simulink model "PrkgClimaEveMgr"  (R2022b) (continued)
== system PrkgClimaEveMgr/PrkgClimaEveMgr_Runnable_sys == (continued)
      settings: EnsureOutportIsVirtual=on
  RtMaxForPrePrkgClima_RtMaxForPrePrkgClima_write : Outport  Port=79
      settings: EnsureOutportIsVirtual=on
  SeatHeatForPrkgClimaEnad_SeatHeatForPrkgClimaEnad_write : Outport  Port=80
      settings: EnsureOutportIsVirtual=on
  SteerWhlHeatgForPrkgClimaEnad_SteerWhlHeatgForPrkgClimaEnad_write : Outport  Port=81
      settings: EnsureOutportIsVirtual=on
  StrtRqrdForDirForPrePrkgClima_StrtRqrdForDirForPrePrkgClima_write : Outport  Port=82
      settings: EnsureOutportIsVirtual=on
  StrtRqrdForPreClng_StrtRqrdForPreClng_write : Outport  Port=83
      settings: EnsureOutportIsVirtual=on
  StrtRqrdForTmrForPrePrkgClima_StrtRqrdForTmrForPrePrkgClima_write : Outport  Port=84
      settings: EnsureOutportIsVirtual=on
  SuspStrtAllwdForPrkgClima_SuspStrtAllwdForPrkgClima_write : Outport  Port=85
      settings: EnsureOutportIsVirtual=on
  TelmClimaExtVentnLvlStrtReq_TelmClimaExtVentnLvlStrtReq_write : Outport  Port=86
      settings: EnsureOutportIsVirtual=on
  TelmClimaExtVentnReqStrtReq_TelmClimaExtVentnReqStrtReq_write : Outport  Port=87
      settings: EnsureOutportIsVirtual=on
  TelmClimaExtVentnTempRangeStrtReq_TelmClimaExtVentnTempRangeStrtReq_write : Outport  Port=88
      settings: EnsureOutportIsVirtual=on
  TelmClimaExtVentnTSpSpclStrtReq_TelmClimaExtVentnTSpSpclStrtReq_write : Outport  Port=89
      settings: EnsureOutportIsVirtual=on
  UpdRtTotForTmrForPrePrkgClima_UpdRtTotForTmrForPrePrkgClima_write : Outport  Port=90
      settings: EnsureOutportIsVirtual=on
  WarnForHybWoU_WarnForHybWoU_write : Outport  Port=91
      settings: EnsureOutportIsVirtual=on
  WarnForHybWoUForTmr_WarnForHybWoUForTmr_write : Outport  Port=92
      settings: EnsureOutportIsVirtual=on
  WarnForPreCdngFctNotAvl_WarnForPreCdngFctNotAvl_write : Outport  Port=93
      settings: EnsureOutportIsVirtual=on
  WarnForTmrPreCdngFctNotAvl_WarnForTmrPreCdngFctNotAvl_write : Outport  Port=94
      settings: EnsureOutportIsVirtual=on
  IntCo2SetStartTime_IntCo2SetStartTime_write : Outport  Port=95
      settings: EnsureOutportIsVirtual=on
  IntCo2SetStopTime_IntCo2SetStopTime_write : Outport  Port=96
      settings: EnsureOutportIsVirtual=on
  RemClimaTempStrtReq_RemClimaTempStrtReq_write : Outport  Port=97
      settings: EnsureOutportIsVirtual=on
  wires:
  AmbTFildForClimaNgcc_AmbTFildForClima_read/1 -> Terminator_1/1
  BookTrvlClimaActvReqfromExsrc_BookTrvlClimaActvReqfromExsrc_read/1 -> Terminator_2/1
  CarTiIntForClima_CarTiIntForClima_read/1 -> Terminator_3/1
  ClimaCfgForCds1_ClimaCfgForCds1_read/1 -> Terminator_4/1
  ClimaCfgForClimaCtrlMan1_ClimaCfgForClimaCtrlMan1_read/1 -> Terminator_5/1
  ClimaCfgForCoolgPreCdn1_ClimaCfgForCoolgPreCdn1_read/1 -> Terminator_6/1
  ClimaCfgForCooltCircForClima1_ClimaCfgForCooltCircForClima1_read/1 -> Terminator_7/1
  ClimaCfgForEcuCooltVlvOutp1_ClimaCfgForEcuCooltVlvOutp1_read/1 -> Terminator_8/1
  ClimaCfgForExtVentnClimaCtrl_ClimaCfgForExtVentnClimaCtrl_read/1 -> Terminator_9/1
  ClimaCfgForFoldCSD_ClimaCfgForFoldCSD_read/1 -> Terminator_10/1
  ClimaCfgForForRemEngStrtPreCdn_ClimaCfgForForRemEngStrtPreCdn_read/1 -> Terminator_11/1
  ClimaCfgForHeatgPreCdnAvl1_ClimaCfgForHeatgPreCdnAvl1_read/1 -> Terminator_12/1
  ClimaCfgForHeatrTyp1_ClimaCfgForHeatrTyp1_read/1 -> Terminator_13/1
  ClimaCfgForHvacHeatrElecFrntPrsnt1_ClimaCfgForHvacHeatrElecFrntPrsnt1_read/1 -> Terminator_14/1
  ClimaCfgForPostCdnAvl1_ClimaCfgForPostCdnAvl1_read/1 -> Terminator_15/1
  ClimaCfgForPreClng1_ClimaCfgForPreClng1_read/1 -> Terminator_16/1
  ClimaCfgForVehElec1_ClimaCfgForVehElec1_read/1 -> Terminator_17/1
  ClimaCfgForVehHyb1_ClimaCfgForVehHyb1_read/1 -> Terminator_18/1
  ClimaCfgForVrntOfVehSysElec1_ClimaCfgForVrntOfVehSysElec1_read/1 -> Terminator_19/1
  ClimaOvrHeatProWarn_ClimaOvrHeatProWarn_read/1 -> Terminator_20/1
  ClimaOvrHeatReqFromExrsc_ClimaOvrHeatReqFromExrsc_read/1 -> Terminator_21/1
  ClimaStrtTiDly_ClimaStrtTiDly_read/1 -> Terminator_22/1
  ClimaTmrSts_ClimaTmrSts_read/1 -> Terminator_23/1
  CmptmtClsd_CmptmtClsd_read/1 -> Terminator_24/1
  COHSts_COHSts_read/1 -> Terminator_25/1
  CooltTForPrkgClima_CooltTForPrkgClima_read/1 -> Terminator_26/1
  DCChrgnHndlSts_DCChrgnHndlSts_read/1 -> Terminator_27/1
  DlyReqForTmrForPrkgClima_DlyReqForTmrForPrkgClima_read/1 -> Terminator_28/1
  DshbTForPrkgClima_DshbTForPrkgClima_read/1 -> Terminator_29/1
  EngT_Ngcc_EngT_read/1 -> Terminator_30/1
  ErsCmdByKeyFromExsrc_ErsCmdByKeyFromExsrc_read/1 -> Terminator_31/1
  ErsRunTimeFromExtSrc_ErsRunTimeFromExtSrc_read/1 -> Terminator_32/1
  HeatrElecNotAvlForTmr_HeatrElecNotAvlForTmr_read/1 -> Terminator_33/1
  HeatrTypSetgForPrkgClima_HeatrTypSetgForPrkgClima_read/1 -> Terminator_34/1
  HeatUpRqrdForPrkgClima_HeatUpRqrdForPrkgClima_read/1 -> Terminator_35/1
  HMIExtVentFanVolReqFromExtSrc_HMIExtVentFanVolReqFromExtSrc_read/1 -> Terminator_36/1
  HMIExtVentonoffReqFromExtSrc_HMIExtVentonoffReqFromExtSrc_read/1 -> Terminator_37/1
  HMIExtVentTSpReqFromExtSrc_HMIExtVentTSpReqFromExtSrc_read/1 -> Terminator_38/1
  HMIExtVentTStsReqFromExtSrc_HMIExtVentTStsReqFromExtSrc_read/1 -> Terminator_39/1
  HvBattSoc_HvBattSoc_read/1 -> Terminator_40/1
  HvSysCnctWoU_HvSysCnctWoU_read/1 -> Terminator_41/1
  HvSysWoU_HvSysWoU_read/1 -> Terminator_42/1
  IntCo2ActvSts_IntCo2ActvSts_read/1 -> Terminator_43/1
  IntCo2fromExsrc_IntCo2fromExsrc_read/1 -> Terminator_44/1
  KeyClimaActv_KeyClimaActv_read/1 -> Terminator_45/1
  LockgCenSts_Ngcc_LockgCenSts_read/1 -> Terminator_46/1
  OnBdChrgrHndlSts_OnBdChrgrHndlSts_read/1 -> Terminator_47/1
  PostDrvgClimaReq_Ngcc_PostDrvgClimaReq_read/1 -> Terminator_48/1
  PreClngRqrdFromExtSrc_PreClngRqrdFromExtSrc_read/1 -> Terminator_49/1
  PrkgClimaDiByCarMod_PrkgClimaDiByCarMod_read/1 -> Terminator_50/1
  PrkgClimaRqrdImdtForBattULo_PrkgClimaRqrdImdtForBattULo_read/1 -> Terminator_51/1
  PrkgClimaRqrdImdtFromActvMgr_PrkgClimaRqrdImdtFromActvMgr_read/1 -> Terminator_52/1
  PrkgClimaRqrdImdtFromExtSrc_PrkgClimaRqrdImdtFromExtSrc_read/1 -> Terminator_53/1
  PrkgClimaRqrdTmrForBattULo_PrkgClimaRqrdTmrForBattULo_read/1 -> Terminator_54/1
  RemClimaFnshd_RemClimaFnshd_read/1 -> Terminator_55/1
  RemClimaReqFromExtSrc_RemClimaReqFromExtSrc_read/1 -> Terminator_56/1
  RemStrtExtnTiReqFromExtSrc_RemStrtExtnTiReqFromExtSrc_read/1 -> Terminator_57/1
  RemStrtHvCtrlReqFromExtSrc_RemStrtHvCtrlReqFromExtSrc_read/1 -> Terminator_58/1
  RemVentFbSts_RemVentFbSts_read/1 -> Terminator_59/1
  RemVentReqfromExsrc_RemVentReqfromExsrc_read/1 -> Terminator_60/1
  ReqForPrkgClima_ReqForPrkgClima_read/1 -> Terminator_61/1
  ReqForSwtHeatrTypFromActvMgr_ReqForSwtHeatrTypFromActvMgr_read/1 -> Terminator_62/1
  RtSdlForPrkgClima_RtSdlForPrkgClima_read/1 -> Terminator_63/1
  SeatHeatgEnadFromHmi_SeatHeatgEnadFromHmi_read/1 -> Terminator_64/1
  SeatHeatgEnadFromTelm_SeatHeatgEnadFromTelm_read/1 -> Terminator_65/1
  SnsrQfOkForPrkgClima_SnsrQfOkForPrkgClima_read/1 -> Terminator_66/1
  SteerWhlHeatgDurgClimaEnadFromExsrc_SteerWhlHeatgDurgClimaEnadFromExsrc_read/1 -> Terminator_67/1
  SteerWhlHeatgEnadFromHmi_SteerWhlHeatgEnadFromHmi_read/1 -> Terminator_68/1
  SteerWhlHeatgEnadFromTelm_SteerWhlHeatgEnadFromTelm_read/1 -> Terminator_69/1
  StrtRemStsFromExsrc_StrtRemStsFromExsrc_read/1 -> Terminator_70/1
  SuspStrtActvForPrkgClima_SuspStrtActvForPrkgClima_read/1 -> Terminator_71/1
  SysDogSlpCountTi_SysDogSlpCountTi_read/1 -> Terminator_72/1
  TelmClimaExtVentnLvlFromExtSrc_TelmClimaExtVentnLvlFromExtSrc_read/1 -> Terminator_73/1
  TelmClimaExtVentnReqFromExtSrc_TelmClimaExtVentnReqFromExtSrc_read/1 -> Terminator_74/1
  TelmClimaExtVentnTempRangeFromExtSrc_TelmClimaExtVentnTempRangeFromExtSrc_read/1 -> Terminator_75/1
  TelmClimaExtVentnTSpSpclFromExtSrc_TelmClimaExtVentnTSpSpclFromExtSrc_read/1 -> Terminator_76/1
  TelmClimaTmrFromExtSrc_TelmClimaTmrFromExtSrc_read/1 -> Terminator_77/1
  TelmSeatDrvrHeatReqFromExsrc_TelmSeatDrvrHeatReqFromExsrc_read/1 -> Terminator_78/1
  TelmSeatDrvVentnReqFromExsrc_TelmSeatDrvVentnReqFromExsrc_read/1 -> Terminator_79/1
  TelmSeatPassHeatReqFromExsrc_TelmSeatPassHeatReqFromExsrc_read/1 -> Terminator_80/1
  TelmSeatPassVentnReqFromExsrc_TelmSeatPassVentnReqFromExsrc_read/1 -> Terminator_81/1
  TelmSeatSecLeHeatReqFromExsrc_TelmSeatSecLeHeatReqFromExsrc_read/1 -> Terminator_82/1
  TelmSeatSecLeVentnReqFromExsrc_TelmSeatSecLeVentnReqFromExsrc_read/1 -> Terminator_83/1
  TelmSeatSecRiHeatReqFromExsrc_TelmSeatSecRiHeatReqFromExsrc_read/1 -> Terminator_84/1
  TelmSeatSecRiVentnReqFromExsrc_TelmSeatSecRiVentnReqFromExsrc_read/1 -> Terminator_85/1
  TForHeatrPreCdngTyp_TForHeatrPreCdngTyp_read/1 -> Terminator_86/1
  TmrForPrkgClima_TmrForPrkgClima_read/1 -> Terminator_87/1
  VehModMngtGlbSafe1_Ngcc_VehModMngtGlbSafe1_read/1 -> Terminator_88/1
  VehSunLoadTot_VehSunLoadTot_read/1 -> Terminator_89/1
  VehSunLoadTotFild_VehSunLoadTotFild_read/1 -> Terminator_90/1
  ClimaCfgForStatrtDevice_ClimaCfgForStartDevice_read/1 -> Terminator_91/1
  PostHeatgRunTiEstimd_PostHeatgRunTiEstimd_read/1 -> Terminator_92/1
  TelmDefrostReqFromExsrc_TelmDefrostReqFromExsrc_read/1 -> Terminator_93/1
  TelmSeatThrdLeHeatReqFromExsrc_TelmSeatThrdLeHeatReqFromExsrc_read/1 -> Terminator_94/1
  TelmSeatThrdLeVentnReqFromExsrc_TelmSeatThrdLeVentnReqFromExsrc_read/1 -> Terminator_95/1
  TelmSeatThrdRiHeatReqFromExsrc_TelmSeatThrdRiHeatReqFromExsrc_read/1 -> Terminator_96/1
  TelmSeatThrdRiVentnReqFromExsrc_TelmSeatThrdRiVentnReqFromExsrc_read/1 -> Terminator_97/1
  TempRangeFromExtSrc_TempRangeFromExtSrc_read/1 -> Terminator_98/1
  CmptmtTFrntRaw_CmptmtTFrntRaw_read/1 -> Terminator_99/1
  BookTrvlClimaActvReq2FromExSrc_BookTrvlClimaActvReq2FromExSrc_read/1 -> Terminator_100/1
  BookTrvlClimaActvReq3FromExSrc_BookTrvlClimaActvReq3FromExSrc_read/1 -> Terminator_101/1
  BookTrvlClimaActvReq4FromExSrc_BookTrvlClimaActvReq4FromExSrc_read/1 -> Terminator_102/1
  BookTrvlClimaActvReq5FromExSrc_BookTrvlClimaActvReq5FromExSrc_read/1 -> Terminator_103/1
  BookTrvlClimaActvReq6FromExSrc_BookTrvlClimaActvReq6FromExSrc_read/1 -> Terminator_104/1
  BookTrvlClimaActvReq7FromExSrc_BookTrvlClimaActvReq7FromExSrc_read/1 -> Terminator_105/1
  BookTrvlClimaFnshd_BookTrvlClimaFnshd_read/1 -> Terminator_106/1
  ClimaActv_Ngcc_ClimaActv_read/1 -> Terminator_107/1
  ClimaCfgForAirMtrlSnsrPrsnt1_ClimaCfgForAirMtrlSnsrPrsnt1_read/1 -> Terminator_108/1
  ClimaCfgForBRAND_ClimaCfgForBRAND_read/1 -> Terminator_109/1
  ClimaCfgForCabinOxgenSupply_ClimaCfgForCabinOxgenSupply_read/1 -> Terminator_110/1
  ClimaCfgForHvacTyp_ClimaCfgForHvacTyp_read/1 -> Terminator_111/1
  ClimaCfgForLifeDetection_ClimaCfgForLifeDetection_read/1 -> Terminator_112/1
  ClimaCfgForPrpsnTyp_ClimaCfgForPrpsnTyp_read/1 -> Terminator_113/1
  ClimaCfgForRFKEY_ClimaCfgForRFKEY_read/1 -> Terminator_114/1
  ClimaCfgForSnsrHumPrsnt1_ClimaCfgForSnsrHumPrsnt1_read/1 -> Terminator_115/1
  ClimaCfgForVehTyp_ClimaCfgForVehTyp_read/1 -> Terminator_116/1
  ClimaWakUpTiDif1FromExSrc_ClimaWakUpTiDif1FromExSrc_read/1 -> Terminator_117/1
  ClimaWakUpTiDif2FromExSrc_ClimaWakUpTiDif2FromExSrc_read/1 -> Terminator_118/1
  ClimaWakUpTiDif3FromExSrc_ClimaWakUpTiDif3FromExSrc_read/1 -> Terminator_119/1
  ClimaWakUpTiDif4FromExSrc_ClimaWakUpTiDif4FromExSrc_read/1 -> Terminator_120/1
  ClimaWakUpTiDif5FromExSrc_ClimaWakUpTiDif5FromExSrc_read/1 -> Terminator_121/1
  ClimaWakUpTiDif6FromExSrc_ClimaWakUpTiDif6FromExSrc_read/1 -> Terminator_122/1
  ClimaWakUpTiDif7FromExSrc_ClimaWakUpTiDif7FromExSrc_read/1 -> Terminator_123/1
  CmptmtAirTEstimdAtRowFirst_CmptmtAirTEstimdAtRowFirst_read/1 -> Terminator_124/1
  CmptmtClngActv_CmptmtClngActv_read/1 -> Terminator_125/1
  CmptmtRelHum_Ngcc_CmptmtRelHum_read/1 -> Terminator_126/1
  CmptmtTRearRaw_CmptmtTRearRaw_read/1 -> Terminator_127/1
  DoorOpenStsForClima_DoorOpenStsForClima_read/1 -> Terminator_128/1
  DoorStsWithOutTrForClima_DoorStsWithOutTrForClima_read/1 -> Terminator_129/1
  EngSt1WdStsFromExsrc_EngSt1WdStsFromExsrc_read/1 -> Terminator_130/1
  FrntHvacBlowerSts_FrntHvacBlowerSts_read/1 -> Terminator_131/1
  GearLvrIndcn_GearLvrIndcn_read/1 -> Terminator_132/1
  GearLvrIndcn_GearLvrIndcn_IsUpdated_read/1 -> Terminator_133/1
  HmiWinterLockCarPrevnIceSetNgcc_HmiWinterLockCarPrevnIceSetNgcc_read/1 -> Terminator_134/1
  HvBattMntnReq_HvBattMntnReq_read/1 -> Terminator_135/1
  HvBattMntnReq_HvBattMntnReq_IsUpdated_read/1 -> Terminator_136/1
  InflateAirSrcSwtNgcc_InflateAirSrcSwtNgcc_read/1 -> Terminator_137/1
  KeyClimaActvFnshd_KeyClimaActvFnshd_read/1 -> Terminator_138/1
  LonglifeBatteryReqNgcc_LonglifeBatteryReqNgcc_read/1 -> Terminator_139/1
  MemReadForPrkgClimaEveMgr_MemReadForPrkgClimaEveMgr_read/1 -> Terminator_140/1
  OGMAirSrcFnshd_OGMAirSrcFnshd_read/1 -> Terminator_141/1
  PrvntWinFrz_PrvntWinFrz_read/1 -> Terminator_142/1
  PrvntWinFrzFnshd_PrvntWinFrzFnshd_read/1 -> Terminator_143/1
  RemClimaFnshd1_RemClimaFnshd1_read/1 -> Terminator_144/1
  RemClimaWarn_RemClimaWarn_read/1 -> Terminator_145/1
  RemOxygenSplyFnshd_RemOxygenSplyFnshd_read/1 -> Terminator_146/1
  RemOxygenSplyFnshd1_RemOxygenSplyFnshd1_read/1 -> Terminator_147/1
  RemOxygenSplyWarn_RemOxygenSplyWarn_read/1 -> Terminator_148/1
  TcsOvrheatdProtnStsNgcc_TcsOvrheatdProtnStsNgcc_read/1 -> Terminator_149/1
  TelmBookClimaReqHmiCmptmtTSpSpclFromExtSrc_TelmBookClimaReqHmiCmptmtTSpSpclFromExtSrc_read/1 -> Terminator_150/1
  TelmBookClimaReqOnOffNoReqFromExtSrc_TelmBookClimaReqOnOffNoReqFromExtSrc_read/1 -> Terminator_151/1
  TelmBookClimaReqTempRangeFromExtSrc_TelmBookClimaReqTempRangeFromExtSrc_read/1 -> Terminator_152/1
  TelmBookClimaTmrFromExtSrc_TelmBookClimaTmrFromExtSrc_read/1 -> Terminator_153/1
  TelmBookSeatHeatClimalReqDrvHeatClimaLvlFromExtSrc_TelmBookSeatHeatClimalReqDrvHeatClimaLvlFromExtSrc_read/1 -> Terminator_154/1
  TelmBookSeatHeatClimalReqPassHeatClimaLvlFromExtSrc_TelmBookSeatHeatClimalReqPassHeatClimaLvlFromExtSrc_read/1 -> Terminator_155/1
  TelmBookSeatHeatClimalReqSecLeHeatClimaLvlFromExtSrc_TelmBookSeatHeatClimalReqSecLeHeatClimaLvlFromExtSrc_read/1 -> Terminator_156/1
  TelmBookSeatHeatClimalReqSecRiHeatClimaLvlFromExtSrc_TelmBookSeatHeatClimalReqSecRiHeatClimaLvlFromExtSrc_read/1 -> Terminator_157/1
  TelmBookSeatHeatClimalReqThrdLeHeatClimaLvlFromExtSrc_TelmBookSeatHeatClimalReqThrdLeHeatClimaLvlFromExtSrc_read/1 -> Terminator_158/1
  TelmBookSeatHeatClimalReqThrdRiHeatClimaLvlFromExtSrc_TelmBookSeatHeatClimalReqThrdRiHeatClimaLvlFromExtSrc_read/1 -> Terminator_159/1
  TelmBookSeatVentnClimalReqDrvVentnClimaLvlFromExtSrc_TelmBookSeatVentnClimalReqDrvVentnClimaLvlFromExtSrc_read/1 -> Terminator_160/1
  TelmBookSeatVentnClimalReqPassVentnClimaLvlFromExtSrc_TelmBookSeatVentnClimalReqPassVentnClimaLvlFromExtSrc_read/1 -> Terminator_161/1
  TelmBookSeatVentnClimalReqSecLeVentnClimaLvlFromExtSrc_TelmBookSeatVentnClimalReqSecLeVentnClimaLvlFromExtSrc_read/1 -> Terminator_162/1
  TelmBookSeatVentnClimalReqSecRiVentnClimaLvlFromExtSrc_TelmBookSeatVentnClimalReqSecRiVentnClimaLvlFromExtSrc_read/1 -> Terminator_163/1
  TelmBookSeatVentnClimalReqThrdLeVentnClimaLvlFromExtSrc_TelmBookSeatVentnClimalReqThrdLeVentnClimaLvlFromExtSrc_read/1 -> Terminator_164/1
  TelmBookSeatVentnClimalReqThrdRiVentnClimaLvlFromExtSrc_TelmBookSeatVentnClimalReqThrdRiVentnClimaLvlFromExtSrc_read/1 -> Terminator_165/1
  TelmBookSteerWhlHeatClimalReqFromExtSrc_TelmBookSteerWhlHeatClimalReqFromExtSrc_read/1 -> Terminator_166/1
  TelmDefrostFnshd_TelmDefrostFnshd_read/1 -> Terminator_167/1
  TelmOxygenReqFromExSrc_TelmOxygenReqFromExSrc_read/1 -> Terminator_168/1
  TelmSeatHeatDrvrFnshd_TelmSeatHeatDrvrFnshd_read/1 -> Terminator_169/1
  TelmSeatHeatPassFnshd_TelmSeatHeatPassFnshd_read/1 -> Terminator_170/1
  TelmSeatHeatSecLeFnshd_TelmSeatHeatSecLeFnshd_read/1 -> Terminator_171/1
  TelmSeatHeatSecRiFnshd_TelmSeatHeatSecRiFnshd_read/1 -> Terminator_172/1
  TelmSeatHeatThrdLeFnshd_TelmSeatHeatThrdLeFnshd_read/1 -> Terminator_173/1
  TelmSeatHeatThrdRiFnshd_TelmSeatHeatThrdRiFnshd_read/1 -> Terminator_174/1
  TelmSeatVentnDrvrFnshd_TelmSeatVentnDrvrFnshd_read/1 -> Terminator_175/1
  TelmSeatVentnPassFnshd_TelmSeatVentnPassFnshd_read/1 -> Terminator_176/1
  TelmSeatVentnSecLeFnshd_TelmSeatVentnSecLeFnshd_read/1 -> Terminator_177/1
  TelmSeatVentnSecRiFnshd_TelmSeatVentnSecRiFnshd_read/1 -> Terminator_178/1
  TelmSeatVentnThrdLeFnshd_TelmSeatVentnThrdLeFnshd_read/1 -> Terminator_179/1
  TelmSeatVentnThrdRiFnshd_TelmSeatVentnThrdRiFnshd_read/1 -> Terminator_180/1
  TelmSteerWhlHeatFnshd_TelmSteerWhlHeatFnshd_read/1 -> Terminator_181/1
  UnLckClimaActvReq_UnLckClimaActvReq_read/1 -> Terminator_182/1
  UnLckHeatActvReq_UnLckHeatActvReq_read/1 -> Terminator_183/1
  VentnForWinOpenGlbActv_VentnForWinOpenGlbActv_read/1 -> Terminator_184/1
  VTMOverTProtectionFnshd_VTMOverTProtectionFnshd_read/1 -> Terminator_185/1
  WarnLvlMsgNgcc_WarnLvlMsgNgcc_read/1 -> Terminator_186/1
  WarnLvlMsgNgcc_WarnLvlMsgNgcc_IsUpdated_read/1 -> Terminator_187/1
  WarnLvlMsgUWB_WarnLvlMsgUWB_read/1 -> Terminator_188/1
  WarnLvlMsgUWB_WarnLvlMsgUWB_IsUpdated_read/1 -> Terminator_189/1
  WinOpenLDACReq_WinOpenLDACReq_read/1 -> Terminator_190/1
  WinOpenLDACReqUWB_WinOpenLDACReqUWB_read/1 -> Terminator_191/1
  WinPosnStsAtDrvrReNgcc_WinPosnStsAtDrvrReNgcc_read/1 -> Terminator_192/1
  WinPosnStsAtPassReNgcc_WinPosnStsAtPassRe_read/1 -> Terminator_193/1
  WinPosnStsDrv_WinPosnStsAtDrv_read/1 -> Terminator_194/1
  WinPosnStsPass_WinPosnStsAtPass_read/1 -> Terminator_195/1
  Ground_1/1 -> HvBattMntnActv_HvBattMntnActv_write/1
  Ground_2/1 -> LDACState_LDACState_write/1
  Ground_3/1 -> MemBookTrvlClimaTi_MemBookTrvlClimaTi_write/1
  Ground_4/1 -> MemSaveFlgForPrkgClimaEveMgr_MemSaveFlgForPrkgClimaEveMgr_write/1
  Ground_5/1(Out1) -> MemWrForPrkgClimaEveMgr_MemWrForPrkgClimaEveMgr_write/1
  Ground_6/1 -> PrkgClimaReqExcpSeat_PrkgClimaReqExcpSeat_write/1
  Ground_7/1 -> PrkgClimaReqPrio_PrkgClimaReqPrio_write/1
  Ground_8/1 -> PrkgOGMAirSrcReq_PrkgOGMAirSrcReq_write/1
  Ground_9/1 -> PrvntWinActvReq_PrvntWinActvReq_write/1
  Ground_10/1 -> RemClimaEgyExsrcReqPlus_RemClimaEgyExsrcReqPlus_write/1
  Ground_11/1 -> RemOxygenSplyReq_RemOxygenSplyReq_write/1
  Ground_12/1 -> TcamClcltTiFb1_TcamClcltTiFb1_write/1
  Ground_13/1 -> TcamClcltTiFb2_TcamClcltTiFb2_write/1
  Ground_14/1 -> TcamClcltTiFb3_TcamClcltTiFb3_write/1
  Ground_15/1 -> TcamClcltTiFb4_TcamClcltTiFb4_write/1
  Ground_16/1 -> TcamClcltTiFb5_TcamClcltTiFb5_write/1
  Ground_17/1 -> TcamClcltTiFb6_TcamClcltTiFb6_write/1
  Ground_18/1 -> TcamClcltTiFb7_TcamClcltTiFb7_write/1
  Ground_19/1 -> TcsOvrheatdProtnReq_TcsOvrheatdProtnReq_write/1
  Ground_20/1 -> TelmBookClimaReqHmiCmptmtTSpSpclStrtReq_TelmBookClimaReqHmiCmptmtTSpSpclStrtReq_write/1
  Ground_21/1 -> TelmBookClimaReqOnOffNoReqStrtReq_TelmBookClimaReqOnOffNoReqStrtReq_write/1
  Ground_22/1 -> TelmBookClimaReqTempRangeStrtReq_TelmBookClimaReqTempRangeStrtReq_write/1
  Ground_23/1 -> TelmBookClimaTmrStrtReq_TelmBookClimaTmrStrtReq_write/1
  Ground_24/1 -> TelmBookSeatHeatClimalReqDrvHeatClimaLvlStrtReq_TelmBookSeatHeatClimalReqDrvHeatClimaLvlStrtReq_write/1
  Ground_25/1 -> TelmBookSeatHeatClimalReqPassHeatClimaLvlStrtReq_TelmBookSeatHeatClimalReqPassHeatClimaLvlStrtReq_write/1
  Ground_26/1 -> TelmBookSeatHeatClimalReqSecLeHeatClimaLvlStrtReq_TelmBookSeatHeatClimalReqSecLeHeatClimaLvlStrtReq_write/1
  Ground_27/1 -> TelmBookSeatHeatClimalReqSecRiHeatClimaLvlStrtReq_TelmBookSeatHeatClimalReqSecRiHeatClimaLvlStrtReq_write/1
  Ground_28/1 -> TelmBookSeatHeatClimalReqThrdLeHeatClimaLvlStrtReq_TelmBookSeatHeatClimalReqThrdLeHeatClimaLvlStrtReq_write/1
  Ground_29/1 -> TelmBookSeatHeatClimalReqThrdRiHeatClimaLvlStrtReq_TelmBookSeatHeatClimalReqThrdRiHeatClimaLvlStrtReq_write/1
  Ground_30/1 -> TelmBookSeatVentnClimalReqDrvVentnClimaLvlStrtReq_TelmBookSeatVentnClimalReqDrvVentnClimaLvlStrtReq_write/1
  Ground_31/1 -> TelmBookSeatVentnClimalReqPassVentnClimaLvlStrtReq_TelmBookSeatVentnClimalReqPassVentnClimaLvlStrtReq_write/1
  Ground_32/1 -> TelmBookSeatVentnClimalReqSecLeVentnClimaLvlStrtReq_TelmBookSeatVentnClimalReqSecLeVentnClimaLvlStrtReq_write/1
  Ground_33/1 -> TelmBookSeatVentnClimalReqSecRiVentnClimaLvlStrtReq_TelmBookSeatVentnClimalReqSecRiVentnClimaLvlStrtReq_write/1
  Ground_34/1 -> TelmBookSeatVentnClimalReqThrdLeVentnClimaLvlStrtReq_TelmBookSeatVentnClimalReqThrdLeVentnClimaLvlStrtReq_write/1
  Ground_35/1 -> TelmBookSeatVentnClimalReqThrdRiVentnClimaLvlStrtReq_TelmBookSeatVentnClimalReqThrdRiVentnClimaLvlStrtReq_write/1
  Ground_36/1 -> TelmBookSteerWhlHeatClimalReqStrtReq_TelmBookSteerWhlHeatClimalReqStrtReq_write/1
  Ground_37/1 -> TelmSeatVentnThrdReq_TelmSeatVentnThrdReq_write/1
  Ground_38/1 -> UnLckClimaActvReqSt_UnLckClimaActvReqSt_write/1
  Ground_39/1 -> UnLckHeatActvReqSt_UnLckHeatActvReqSt_write/1
  Ground_40/1 -> ActvRqrdForPostPrkgClima_ActvRqrdForPostPrkgClima_write/1
  Ground_41/1 -> ClimaOvrHeatProWarnIni_ClimaOvrHeatProWarnIni_write/1
  Ground_42/1 -> ClimaOvrHeatStrtReq_ClimaOvrHeatStrtReq_write/1
  Ground_43/1 -> ClimaStrtTi_ClimaStrtTi_write/1
  Ground_44/1 -> CSDUsgChgeOffReq_CSDUsgChgeOffReq_write/1
  Ground_45/1 -> CSDUsgChgeOnReq_CSDUsgChgeOnReq_write/1
  Ground_46/1 -> EcuDogKeepActv_EcuDogKeepActv_write/1
  Ground_47/1 -> EcuDogSlpReq_EcuDogSlpReq_write/1
  Ground_48/1 -> EcuDogSlpSetTi_EcuDogSlpSetTi_write/1
  Ground_49/1 -> ErsCmdByKeyStrtReq_ErsCmdByKeyStrtReq_write/1
  Ground_50/1 -> ErsRunTimelStrtReq_ErsRunTimelStrtReq_write/1
  Ground_51/1 -> HeatrTypForPrkgClima_HeatrTypForPrkgClima_write/1
  Ground_52/1 -> HMIExtVentFanVolReqStrtReq_HMIExtVentFanVolReqStrtReq_write/1
  Ground_53/1 -> HMIExtVentonoffReqStrtReq_HMIExtVentonoffReqStrtReq_write/1
  Ground_54/1 -> HMIExtVentTSpReqStrtReq_HMIExtVentTSpReqStrtReq_write/1
  Ground_55/1 -> HMIExtVentTStsReqStrtReq_HMIExtVentTStsReqStrtReq_write/1
  Ground_56/1 -> HvBattForPrkgClima_HvBattForPrkgClima_write/1
  Ground_57/1 -> IntCo2MontrStrtReq_IntCo2MontrStrtReq_write/1
  Ground_58/1 -> NetReqFromPrkgClimaEveMgr_NetReqFromPrkgClimaEveMgr_write/1
  Ground_59/1 -> ObsvrActvnReqFromPrkgClima_ObsvrActvnReqFromPrkgClima_write/1
  Ground_60/1 -> OffRqrdForPrkgClima_OffRqrdForPrkgClima_write/1
  Ground_61/1 -> OffRqrdForRemClima_OffRqrdForRemClima_write/1
  Ground_62/1 -> PostDrvgClimaAvl_Ngcc_PostDrvgClimaAvl_write/1
  Ground_63/1 -> PrePrkgClimaNotAvlForHvSysWoU_PrePrkgClimaNotAvlForHvSysWoU_write/1
  Ground_64/1 -> PrkgCDSAvl_PrkgCDSAvl_write/1
  Ground_65/1 -> PrkgClimaAllwdForBattULo_PrkgClimaAllwdForBattULo_write/1
  Ground_66/1 -> PrkgClimaAllwdForSwtHeatrTyp_PrkgClimaAllwdForSwtHeatrTyp_write/1
  Ground_67/1 -> RemClimaallExsrcReq_RemClimaallExsrcReq_write/1
  Ground_68/1 -> RemClimaEgyExsrcReq_RemClimaEgyExsrcReq_write/1
  Ground_69/1 -> RemClimaTmrStrtReq_RemClimaTmrStrtReq_write/1
  Ground_70/1 -> RemStrtExtnTiStrtReq_RemStrtExtnTiStrtReq_write/1
  Ground_71/1 -> RemStrtHvCtrlStrtReq_RemStrtHvCtrlStrtReq_write/1
  Ground_72/1 -> RemVentReqStrtReq_RemVentReqStrtReq_write/1
  Ground_73/1 -> ReqForSwtHeatrTypFromEveMgr_ReqForSwtHeatrTypFromEveMgr_write/1
  Ground_74/1 -> RtBasSdlForTmrPrePrkgClima_RtBasSdlForTmrPrePrkgClima_write/1
  Ground_75/1 -> RtCmftForImdtPrePrkgClima_RtCmftForImdtPrePrkgClima_write/1
  Ground_76/1 -> RtCmftForPostPrkgClima_RtCmftForPostPrkgClima_write/1
  Ground_77/1 -> RtCmftForPreClng_RtCmftForPreClng_write/1
  Ground_78/1 -> RtCmftForTmrPrePrkgClima_RtCmftForTmrPrePrkgClima_write/1
  Ground_79/1 -> RtMaxForPrePrkgClima_RtMaxForPrePrkgClima_write/1
  Ground_80/1 -> SeatHeatForPrkgClimaEnad_SeatHeatForPrkgClimaEnad_write/1
  Ground_81/1 -> SteerWhlHeatgForPrkgClimaEnad_SteerWhlHeatgForPrkgClimaEnad_write/1
  Ground_82/1 -> StrtRqrdForDirForPrePrkgClima_StrtRqrdForDirForPrePrkgClima_write/1
  Ground_83/1 -> StrtRqrdForPreClng_StrtRqrdForPreClng_write/1
  Ground_84/1 -> StrtRqrdForTmrForPrePrkgClima_StrtRqrdForTmrForPrePrkgClima_write/1
  Ground_85/1 -> SuspStrtAllwdForPrkgClima_SuspStrtAllwdForPrkgClima_write/1
  Ground_86/1 -> TelmClimaExtVentnLvlStrtReq_TelmClimaExtVentnLvlStrtReq_write/1
  Ground_87/1 -> TelmClimaExtVentnReqStrtReq_TelmClimaExtVentnReqStrtReq_write/1
  Ground_88/1 -> TelmClimaExtVentnTempRangeStrtReq_TelmClimaExtVentnTempRangeStrtReq_write/1
  Ground_89/1 -> TelmClimaExtVentnTSpSpclStrtReq_TelmClimaExtVentnTSpSpclStrtReq_write/1
  Ground_90/1 -> UpdRtTotForTmrForPrePrkgClima_UpdRtTotForTmrForPrePrkgClima_write/1
  Ground_91/1 -> WarnForHybWoU_WarnForHybWoU_write/1
  Ground_92/1 -> WarnForHybWoUForTmr_WarnForHybWoUForTmr_write/1
  Ground_93/1 -> WarnForPreCdngFctNotAvl_WarnForPreCdngFctNotAvl_write/1
  Ground_94/1 -> WarnForTmrPreCdngFctNotAvl_WarnForTmrPreCdngFctNotAvl_write/1
  Ground_95/1 -> IntCo2SetStartTime_IntCo2SetStartTime_write/1
  Ground_96/1 -> IntCo2SetStopTime_IntCo2SetStopTime_write/1
  Ground_97/1 -> RemClimaTempStrtReq_RemClimaTempStrtReq_write/1

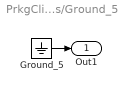
== system PrkgClimaEveMgr/PrkgClimaEveMgr_Runnable_sys/Ground_5 ==
  Ground_5 : Ground
  Out1 : Outport  OutDataTypeStr=Bus: MemRecForPrkgClimaEveMgr  PortDimensions=1
  wires:
  Ground_5/1 -> Out1/1
